simulink model "HvacActrPosnReqFrnt"  (R2022b)
settings: Solver=FixedStepDiscrete  StartTime=0.0  StopTime=10.0  FixedStep=0.2  RelTol=1e-3
(part 2 of 2)

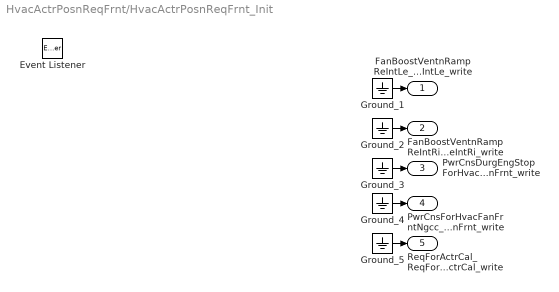
== system HvacActrPosnReqFrnt/HvacActrPosnReqFrnt_Init ==
  Event Listener : EventListener  EventName=reset
  Ground_{1..5} (5 alike) : Ground
  FanBoostVentnRampReIntLe_FanBoostVentnRampReIntLe_write : Outport
  FanBoostVentnRampReIntRi_FanBoostVentnRampReIntRi_write : Outport  Port=2
  PwrCnsDurgEngStopForHvacFanFrnt_PwrCnsDurgEngStopForHvacFanFrnt_write : Outport  Port=3
  PwrCnsForHvacFanFrntNgcc_PwrCnsForHvacFanFrnt_write : Outport  Port=4
  ReqForActrCal_ReqForActrCal_write : Outport  Port=5
  wires:
  Ground_1/1 -> FanBoostVentnRampReIntLe_FanBoostVentnRampReIntLe_write/1
  Ground_2/1 -> FanBoostVentnRampReIntRi_FanBoostVentnRampReIntRi_write/1
  Ground_3/1 -> PwrCnsDurgEngStopForHvacFanFrnt_PwrCnsDurgEngStopForHvacFanFrnt_write/1
  Ground_4/1 -> PwrCnsForHvacFanFrntNgcc_PwrCnsForHvacFanFrnt_write/1
  Ground_5/1 -> ReqForActrCal_ReqForActrCal_write/1

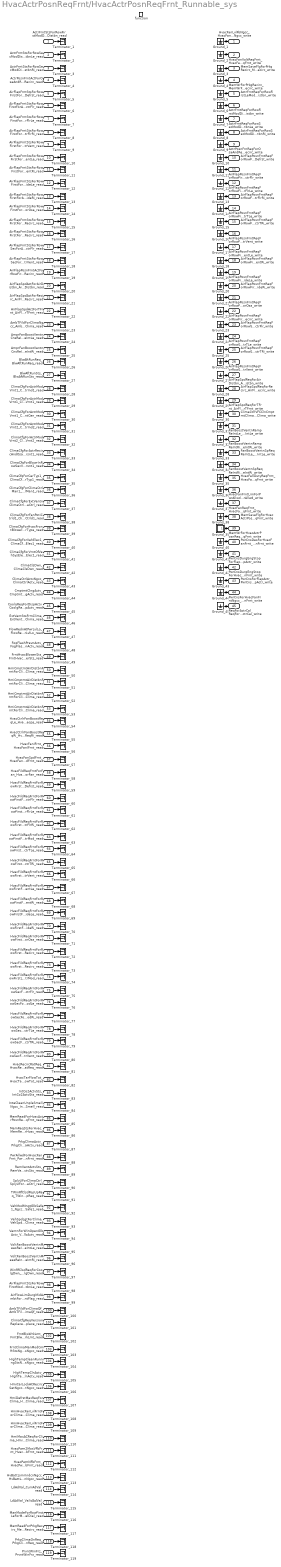
== system HvacActrPosnReqFrnt/HvacActrPosnReqFrnt_Runnable_sys ==
  ActrFrntStsForRowFirstModDistbn_ActrFrntStsForRowFirstModDistbn_read : Inport
  ActrFrntStsForRowSecModDistbnLe_ActrFrntStsForRowSecModDistbnLe_read : Inport  Port=2
  ActrFrntStsForRowSecModDistbnRi_ActrFrntStsForRowSecModDistbnRi_read : Inport  Port=3
  ActrPosnFrntActForOsaAndRecirc_ActrPosnFrntActForOsaAndRecirc_read : Inport  Port=4
  AirFlapFrntStsForRowFirstForActrDefrst_AirFlapFrntStsForRowFirstForActrDefrst_read : Inport  Port=5
  AirFlapFrntStsForRowFirstForActrFlr_AirFlapFrntStsForRowFirstForActrFlr_read : Inport  Port=6
  AirFlapFrntStsForRowFirstForActrFlrLe_AirFlapFrntStsForRowFirstForActrFlrLe_read : Inport  Port=7
  AirFlapFrntStsForRowFirstForActrFlrRi_AirFlapFrntStsForRowFirstForActrFlrRi_read : Inport  Port=8
  AirFlapFrntStsForRowFirstForActrVent_AirFlapFrntStsForRowFirstForActrVent_read : Inport  Port=9
  AirFlapFrntStsForRowFirstForActrVentLe_AirFlapFrntStsForRowFirstForActrVentLe_read : Inport  Port=10
  AirFlapFrntStsForRowFirstForActrVentRi_AirFlapFrntStsForRowFirstForActrVentRi_read : Inport  Port=11
  AirFlapFrntStsForRowFirstForActrVentSideLe_AirFlapFrntStsForRowFirstForActrVentSideLe_read : Inport  Port=12
  AirFlapFrntStsForRowFirstForActrVentSideRi_AirFlapFrntStsForRowFirstForActrVentSideRi_read : Inport  Port=13
  AirFlapFrntStsForRowFirstForOsa_AirFlapFrntStsForRowFirstForOsa_read : Inport  Port=14
  AirFlapFrntStsForRowFirstForOsaAndRecirc_AirFlapFrntStsForRowFirstForOsaAndRecirc_read : Inport  Port=15
  AirFlapFrntStsForRowFirstForRecirc_AirFlapFrntStsForRowFirstForRecirc_read : Inport  Port=16
  AirFlapFrntStsForRowSecForActrFlr_AirFlapFrntStsForRowSecForActrFlr_read : Inport  Port=17
  AirFlapFrntStsForRowSecForActrVent_AirFlapFrntStsForRowSecForActrVent_read : Inport  Port=18
  AirFlapPosnFrntActForRowFirstForRecirc_AirFlapPosnFrntActForRowFirstForRecirc_read : Inport  Port=19
  AirFlapSpdActForAirDistbn_AirFlapSpdActForAirDistbn_read : Inport  Port=20
  AirFlapSpdActForRecirc_AirFlapSpdActForRecirc_read : Inport  Port=21
  AirFlapSpdActForTFrnt_AirFlapSpdActForTFrnt_read : Inport  Port=22
  AmbTFildForClimaNgcc_AmbTFildForClima_read : Inport  Port=23
  AmprFanBoostVentnCnsReIntLe_AmprFanBoostVentnCnsReIntLe_read : Inport  Port=24
  AmprFanBoostVentnCnsReIntRi_AmprFanBoostVentnCnsReIntRi_read : Inport  Port=25
  BlwAftRunReq_BlwAftRunReq_read : Inport  Port=26
  BlwAftRunSts_BlwAftRunSts_read : Inport  Port=27
  ClimaCfgForActrModVrnt1_ClimaCfgForActrInd1_read : Inport  Port=28
  ClimaCfgForActrModVrnt1_ClimaCfgForActrModVrnt1_read : Inport  Port=29
  ClimaCfgForActrModVrnt1_ClimaCfgForActrModVrntCen_read : Inport  Port=30
  ClimaCfgForActrModVrnt2_ClimaCfgForActrInd2_read : Inport  Port=31
  ClimaCfgForActrModVrnt2_ClimaCfgForActrModVrnt2_read : Inport  Port=32
  ClimaCfgForActrRecircAndOsaCmnPrsnt1_ClimaCfgForActrRecircAndOsaCmnPrsnt1_read : Inport  Port=33
  ClimaCfgForBlowrInRowSecVentSidePrsnt1_ClimaCfgForBlowrInRowSecVentSidePrsnt1_read : Inport  Port=34
  ClimaCfgForCarTyp1_ClimaCfgForCarTyp1_read : Inport  Port=35
  ClimaCfgForClimaCtrlMan1_ClimaCfgForClimaCtrlMan1_read : Inport  Port=36
  ClimaCfgForExtVentnClimaCtrl_ClimaCfgForExtVentnClimaCtrl_read : Inport  Port=37
  ClimaCfgForFanPotiCtrld1_ClimaCfgForFanPotiCtrld1_read : Inport  Port=38
  ClimaCfgForHvacFrontBlowerType_ClimaCfgForHvacFrontBlowerType_read : Inport  Port=39
  ClimaCfgForVehElec1_ClimaCfgForVehElec1_read : Inport  Port=40
  ClimaCfgForVrntOfVehSysElec1_ClimaCfgForVrntOfVehSysElec1_read : Inport  Port=41
  ClimaClsDwn_ClimaClsDwn_read : Inport  Port=42
  ClimaCtrlActvNgcc_ClimaCtrlActv_read : Inport  Port=43
  CmptmtClngActv_CmptmtClngActv_read : Inport  Port=44
  CoolgReqForDispActv_CoolgReqForDispActv_read : Inport  Port=45
  ExtVentStsFrmClima_ExtVentStsFrmClima_read : Inport  Port=46
  FlowRednAtPwrLvlLo_FlowRednAtPwrLvlLo_read : Inport  Port=47
  FogFlashPrevnActv_FogFlashPrevnActv_read : Inport  Port=48
  FrntHvacBlowerSts_FrntHvacBlowerSts_read : Inport  Port=49
  HmiCmptmtAirDistbnFrntForClima_HmiCmptmtAirDistbnFrntForClima_read : Inport  Port=50
  HmiCmptmtAirDistbnFrntForClima_HmiCmptmtAirDistbnFrntRiForClima_read : Inport  Port=51
  HmiCmptmtAirDistbnFrntForClima_HmiCmptmtAirDistbnReForClima_read : Inport  Port=52
  HmiCmptmtAirDistbnFrntForClima_HmiCmptmtAirDistbnReRiForClima_read : Inport  Port=53
  HvacCtrlrFanBoostReqLe_HvacCtrlrFanBoostReqLe_read : Inport  Port=54
  HvacCtrlrFanBoostReqRi_HvacCtrlrFanBoostReqRi_read : Inport  Port=55
  HvacFanIFrnt_HvacFanIFrnt_read : Inport  Port=56
  HvacFanSpdFrnt_HvacFanSpdFrnt_read : Inport  Port=57
  HvacFildReqFrntForFan_HvacFildReqFrntForFan_read : Inport  Port=58
  HvacFildReqFrntForRowFirstForActrDefrst_HvacFildReqFrntForRowFirstForActrDefrst_read : Inport  Port=59
  HvacFildReqFrntForRowFirstForActrFlr_HvacFildReqFrntForRowFirstForActrFlr_read : Inport  Port=60
  HvacFildReqFrntForRowFirstForActrFlr_HvacFildReqFrntForRowFirstForActrFlrLe_read : Inport  Port=61
  HvacFildReqFrntForRowFirstForActrFlr_HvacFildReqFrntForRowFirstForActrFlrRi_read : Inport  Port=62
  HvacFildReqFrntForRowFirstForActrMod_HvacFildReqFrntForRowFirstForActrMod_read : Inport  Port=63
  HvacFildReqFrntForRowFirstForActrTLe_HvacFildReqFrntForRowFirstForActrTLe_read : Inport  Port=64
  HvacFildReqFrntForRowFirstForActrTRi_HvacFildReqFrntForRowFirstForActrTRi_read : Inport  Port=65
  HvacFildReqFrntForRowFirstForActrVent_HvacFildReqFrntForRowFirstForActrVent_read : Inport  Port=66
  HvacFildReqFrntForRowFirstForActrVent_HvacFildReqFrntForRowFirstForActrVentLe_read : Inport  Port=67
  HvacFildReqFrntForRowFirstForActrVent_HvacFildReqFrntForRowFirstForActrVentRi_read : Inport  Port=68
  HvacFildReqFrntForRowFirstForActrVent_HvacFildReqFrntForRowFirstForActrVentSideLe_read : Inport  Port=69
  HvacFildReqFrntForRowFirstForActrVent_HvacFildReqFrntForRowFirstForActrVentSideRi_read : Inport  Port=70
  HvacFildReqFrntForRowFirstForOsa_HvacFildReqFrntForRowFirstForOsa_read : Inport  Port=71
  HvacFildReqFrntForRowFirstForOsaAndRecirc_HvacFildReqFrntForRowFirstForOsaAndRecirc_read : Inport  Port=72
  HvacFildReqFrntForRowFirstForRecirc_HvacFildReqFrntForRowFirstForRecirc_read : Inport  Port=73
  HvacFildReqFrntForRowFirstLeForActrMod_HvacFildReqFrntForRowFirstLeForActrMod_read : Inport  Port=74
  HvacFildReqFrntForRowSecForActrFlr_HvacFildReqFrntForRowSecForActrFlr_read : Inport  Port=75
  HvacFildReqFrntForRowSecForActrModLe_HvacFildReqFrntForRowSecForActrModLe_read : Inport  Port=76
  HvacFildReqFrntForRowSecForActrModRi_HvacFildReqFrntForRowSecForActrModRi_read : Inport  Port=77
  HvacFildReqFrntForRowSecForActrTLe_HvacFildReqFrntForRowSecForActrTLe_read : Inport  Port=78
  HvacFildReqFrntForRowSecForActrTRi_HvacFildReqFrntForRowSecForActrTRi_read : Inport  Port=79
  HvacFildReqFrntForRowSecForActrVent_HvacFildReqFrntForRowSecForActrVent_read : Inport  Port=80
  HvacRecircRatReq_HvacRecircRatReq_read : Inport  Port=81
  HvacTarFlowTot_HvacTarFlowTot_read : Inport  Port=82
  IntCo2ActvSts_IntCo2ActvSts_read : Inport  Port=83
  InteCleanUnpleSmell_Ngcc_InteCleanUnpleSmell_read : Inport  Port=84
  MemReadForHvacActrPosnReqFrnt_MemReadForHvacActrPosnReqFrnt_read : Inport  Port=85
  MemReqStsForHvac_MemReqStsForHvac_read : Inport  Port=86
  PrkgClimaActv_PrkgClimaActv_read : Inport  Port=87
  PwrAllwdForHvacFanFrnt_PwrAllwdForHvacFanFrnt_read : Inport  Port=88
  RemVentActvSts_RemVentActvSts_read : Inport  Port=89
  SplyUForClimaCtrl_SplyUForClimaCtrl_read : Inport  Port=90
  TWinRfClsdPopUpReq_TWinRfClsdPopUpReq_read : Inport  Port=91
  VehModMngtGlbSafe1_Ngcc_VehModMngtGlbSafe1_read : Inport  Port=92
  VehSpdLgtForClima_VehSpdLgtForClima_read : Inport  Port=93
  VentnForWinOpenGlbActv_VentnForWinOpenGlbActv_read : Inport  Port=94
  VoltFanBoostVentnMeasReIntLe_VoltFanBoostVentnMeasReIntLe_read : Inport  Port=95
  VoltFanBoostVentnMeasReIntRi_VoltFanBoostVentnMeasReIntRi_read : Inport  Port=96
  WinRfClsdReqForCoolgDwn_WinRfClsdReqForCoolgDwn_read : Inport  Port=97
  AirFlapFrntStsForRowFirstModDistbnLe_AirFlapFrntStsForRowFirstModDistbnLe_read : Inport  Port=98
  AirFlowLimDurgMidAmbtForNVHForHVACFrntFlag_AirFlowLimDurgMidAmbtForNVHForHVACFrntFlag_read : Inport  Port=99
  AmbTFildForClimaQf_AmbTFildForClimaQf_read : Inport  Port=100
  ClimaCfgReqVersionReplace_ClimaCfgReqVersionReplace_read : Inport  Port=101
  FrntBlwWrkLmt_FrntBlwWrkLmt_read : Inport  Port=102
  FrntClimaManModCmftStsNgcc_FrntClimaManModCmftStsNgcc_read : Inport  Port=103
  HighTempCleanRunningStsNgcc_HighTempCleanRunningStsNgcc_read : Inport  Port=104
  HighTempClnActv_HighTempClnActv_read : Inport  Port=105
  HmiCarLockACRecircSetNgcc_HmiCarLockACRecircSetNgcc_read : Inport  Port=106
  HmiDefrstMaxReqForClima_HmiDefrstMaxReqForClima_read : Inport  Port=107
  HmiHvacFanLvlFrntForClima_HmiHvacFanLvlFrntForClima_read : Inport  Port=108
  HmiHvacFanLvlFrntForClima_HmiHvacFanLvlFrntRiForClima_read : Inport  Port=109
  HmiMaxACReqForClima_HmiMaxACReqForClima_read : Inport  Port=110
  HvacPwm2MosVFbFrnt_HvacPwm2MosVFbFrnt_read : Inport  Port=111
  HvacPwmNFbFrnt_HvacPwmNFbFrnt_read : Inport  Port=112
  HvBattLimnIndcnNgcc_HvBattLimnIndcnNgcc_read : Inport  Port=113
  LdAdVal_CurrAdVal_read : Inport  Port=114
  LdAdVal_ValidAdVal_read : Inport  Port=115
  ManModeForRowFirstLeForModelDial_ManModeForRowFirstLeForModelDial_read : Inport  Port=116
  MemReadForPrkgRecirc_MemReadForPrkgRecirc_read : Inport  Port=117
  PrkgClimaOnReq_PrkgClimaOnReq_read : Inport  Port=118
  PrvntWinFrz_PrvntWinFrz_read : Inport  Port=119
  function : TriggerPort  TriggerType=function-call  VariantControl=(inherit)  SampleTimeType=periodic  SampleTime=-1
  Ground_{1..38,40..45} (44 alike) : Ground
  Ground_39 : Subsystem
  Terminator_{1..119} (119 alike) : Terminator
  HvacFanLvlFbNgcc_HvacFanLvlFbNgcc_write : Outport
      settings: EnsureOutportIsVirtual=on
  HvacFanVoltReqFrnt_HvacFanVoltReqFrnt_write : Outport  Port=2
      settings: EnsureOutportIsVirtual=on
  MemSaveFlgForPrkgRecirc_MemSaveFlgForPrkgRecirc_write : Outport  Port=3
      settings: EnsureOutportIsVirtual=on
  MemWrForPrkgRecirc_MemWrForPrkgRecirc_write : Outport  Port=4
      settings: EnsureOutportIsVirtual=on
  ActrFrntReqForRowFirstLeModDistbn_ActrFrntReqForRowFirstLeModDistbn_write : Outport  Port=5
      settings: EnsureOutportIsVirtual=on
  ActrFrntReqForRowFirstModDistbn_ActrFrntReqForRowFirstModDistbn_write : Outport  Port=6
      settings: EnsureOutportIsVirtual=on
  ActrFrntReqForRowSecModDistbnLe_ActrFrntReqForRowSecModDistbnLe_write : Outport  Port=7
      settings: EnsureOutportIsVirtual=on
  ActrFrntReqForRowSecModDistbnRi_ActrFrntReqForRowSecModDistbnRi_write : Outport  Port=8
      settings: EnsureOutportIsVirtual=on
  ActrPosnFrnReqForOsaAndRecirc_ActrPosnFrnReqForOsaAndRecirc_write : Outport  Port=9
      settings: EnsureOutportIsVirtual=on
  AirFlapPosnFrntReqForRowFirstForActrDefrst_AirFlapPosnFrntReqForRowFirstForActrDefrst_write : Outport  Port=10
      settings: EnsureOutportIsVirtual=on
  AirFlapPosnFrntReqForRowFirstForActrFlr_AirFlapPosnFrntReqForRowFirstForActrFlr_write : Outport  Port=11
      settings: EnsureOutportIsVirtual=on
  AirFlapPosnFrntReqForRowFirstForActrFlrLe_AirFlapPosnFrntReqForRowFirstForActrFlrLe_write : Outport  Port=12
      settings: EnsureOutportIsVirtual=on
  AirFlapPosnFrntReqForRowFirstForActrFlrRi_AirFlapPosnFrntReqForRowFirstForActrFlrRi_write : Outport  Port=13
      settings: EnsureOutportIsVirtual=on
  AirFlapPosnFrntReqForRowFirstForActrTLe_AirFlapPosnFrntReqForRowFirstForActrTLe_write : Outport  Port=14
      settings: EnsureOutportIsVirtual=on
  AirFlapPosnFrntReqForRowFirstForActrTRi_AirFlapPosnFrntReqForRowFirstForActrTRi_write : Outport  Port=15
      settings: EnsureOutportIsVirtual=on
  AirFlapPosnFrntReqForRowFirstForActrVent_AirFlapPosnFrntReqForRowFirstForActrVent_write : Outport  Port=16
      settings: EnsureOutportIsVirtual=on
  AirFlapPosnFrntReqForRowFirstForActrVentLe_AirFlapPosnFrntReqForRowFirstForActrVentLe_write : Outport  Port=17
      settings: EnsureOutportIsVirtual=on
  AirFlapPosnFrntReqForRowFirstForActrVentRi_AirFlapPosnFrntReqForRowFirstForActrVentRi_write : Outport  Port=18
      settings: EnsureOutportIsVirtual=on
  AirFlapPosnFrntReqForRowFirstForActrVentSideLe_AirFlapPosnFrntReqForRowFirstForActrVentSideLe_write : Outport  Port=19
      settings: EnsureOutportIsVirtual=on
  AirFlapPosnFrntReqForRowFirstForActrVentSideRi_AirFlapPosnFrntReqForRowFirstForActrVentSideRi_write : Outport  Port=20
      settings: EnsureOutportIsVirtual=on
  AirFlapPosnFrntReqForRowFirstForOsa_AirFlapPosnFrntReqForRowFirstForOsa_write : Outport  Port=21
      settings: EnsureOutportIsVirtual=on
  AirFlapPosnFrntReqForRowFirstForRecirc_AirFlapPosnFrntReqForRowFirstForRecirc_write : Outport  Port=22
      settings: EnsureOutportIsVirtual=on
  AirFlapPosnFrntReqForRowSecForActrFlr_AirFlapPosnFrntReqForRowSecForActrFlr_write : Outport  Port=23
      settings: EnsureOutportIsVirtual=on
  AirFlapPosnFrntReqForRowSecForActrTLe_AirFlapPosnFrntReqForRowSecForActrTLe_write : Outport  Port=24
      settings: EnsureOutportIsVirtual=on
  AirFlapPosnFrntReqForRowSecForActrTRi_AirFlapPosnFrntReqForRowSecForActrTRi_write : Outport  Port=25
      settings: EnsureOutportIsVirtual=on
  AirFlapPosnFrntReqForRowSecForActrVent_AirFlapPosnFrntReqForRowSecForActrVent_write : Outport  Port=26
      settings: EnsureOutportIsVirtual=on
  AirFlapSpdReqForAirDistbn_AirFlapSpdReqForAirDistbn_write : Outport  Port=27
      settings: EnsureOutportIsVirtual=on
  AirFlapSpdReqForRecirc_AirFlapSpdReqForRecirc_write : Outport  Port=28
      settings: EnsureOutportIsVirtual=on
  AirFlapSpdReqForTFrnt_AirFlapSpdReqForTFrnt_write : Outport  Port=29
      settings: EnsureOutportIsVirtual=on
  ClimaCtrlVFCClnCmptmtClima_ClimaCtrlVFCClnCmptmtClima_write : Outport  Port=30
      settings: EnsureOutportIsVirtual=on
  FanBoostVentnRampReIntLe_FanBoostVentnRampReIntLe_write : Outport  Port=31
      settings: EnsureOutportIsVirtual=on
  FanBoostVentnRampReIntRi_FanBoostVentnRampReIntRi_write : Outport  Port=32
      settings: EnsureOutportIsVirtual=on
  FanBoostVentnSpReqReIntLe_FanBoostVentnSpReqReIntLe_write : Outport  Port=33
      settings: EnsureOutportIsVirtual=on
  FanBoostVentnSpReqReIntRi_FanBoostVentnSpReqReIntRi_write : Outport  Port=34
      settings: EnsureOutportIsVirtual=on
  HvacFanDutyReqFrnt_HvacFanDutyReqFrnt_write : Outport  Port=35
      settings: EnsureOutportIsVirtual=on
  HvacFanFrntLimForPwrAllwd_HvacFanFrntLimForPwrAllwd_write : Outport  Port=36
      settings: EnsureOutportIsVirtual=on
  HvacFanReqFrnt_HvacFanReqFrnt_write : Outport  Port=37
      settings: EnsureOutportIsVirtual=on
  MemSaveFlgForHvacActrPosnReqFrnt_MemSaveFlgForHvacActrPosnReqFrnt_write : Outport  Port=38
      settings: EnsureOutportIsVirtual=on
  MemWrForHvacActrPosnReqFrnt_MemWrForHvacActrPosnReqFrnt_write : Outport  Port=39
      settings: EnsureOutportIsVirtual=on
  PwrCnsDesForHvacFanFrnt_PwrCnsDesForHvacFanFrnt_write : Outport  Port=40
      settings: EnsureOutportIsVirtual=on
  PwrCnsDurgEngStopForFlapActr_PwrCnsDurgEngStopForFlapActr_write : Outport  Port=41
      settings: EnsureOutportIsVirtual=on
  PwrCnsDurgEngStopForHvacFanFrnt_PwrCnsDurgEngStopForHvacFanFrnt_write : Outport  Port=42
      settings: EnsureOutportIsVirtual=on
  PwrCnsForFlapActr_PwrCnsForFlapActr_write : Outport  Port=43
      settings: EnsureOutportIsVirtual=on
  PwrCnsForHvacFanFrntNgcc_PwrCnsForHvacFanFrnt_write : Outport  Port=44
      settings: EnsureOutportIsVirtual=on
  ReqForActrCal_ReqForActrCal_write : Outport  Port=45
      settings: EnsureOutportIsVirtual=on
  wires:
  ActrFrntStsForRowFirstModDistbn_ActrFrntStsForRowFirstModDistbn_read/1 -> Terminator_1/1
  ActrFrntStsForRowSecModDistbnLe_ActrFrntStsForRowSecModDistbnLe_read/1 -> Terminator_2/1
  ActrFrntStsForRowSecModDistbnRi_ActrFrntStsForRowSecModDistbnRi_read/1 -> Terminator_3/1
  ActrPosnFrntActForOsaAndRecirc_ActrPosnFrntActForOsaAndRecirc_read/1 -> Terminator_4/1
  AirFlapFrntStsForRowFirstForActrDefrst_AirFlapFrntStsForRowFirstForActrDefrst_read/1 -> Terminator_5/1
  AirFlapFrntStsForRowFirstForActrFlr_AirFlapFrntStsForRowFirstForActrFlr_read/1 -> Terminator_6/1
  AirFlapFrntStsForRowFirstForActrFlrLe_AirFlapFrntStsForRowFirstForActrFlrLe_read/1 -> Terminator_7/1
  AirFlapFrntStsForRowFirstForActrFlrRi_AirFlapFrntStsForRowFirstForActrFlrRi_read/1 -> Terminator_8/1
  AirFlapFrntStsForRowFirstForActrVent_AirFlapFrntStsForRowFirstForActrVent_read/1 -> Terminator_9/1
  AirFlapFrntStsForRowFirstForActrVentLe_AirFlapFrntStsForRowFirstForActrVentLe_read/1 -> Terminator_10/1
  AirFlapFrntStsForRowFirstForActrVentRi_AirFlapFrntStsForRowFirstForActrVentRi_read/1 -> Terminator_11/1
  AirFlapFrntStsForRowFirstForActrVentSideLe_AirFlapFrntStsForRowFirstForActrVentSideLe_read/1 -> Terminator_12/1
  AirFlapFrntStsForRowFirstForActrVentSideRi_AirFlapFrntStsForRowFirstForActrVentSideRi_read/1 -> Terminator_13/1
  AirFlapFrntStsForRowFirstForOsa_AirFlapFrntStsForRowFirstForOsa_read/1 -> Terminator_14/1
  AirFlapFrntStsForRowFirstForOsaAndRecirc_AirFlapFrntStsForRowFirstForOsaAndRecirc_read/1 -> Terminator_15/1
  AirFlapFrntStsForRowFirstForRecirc_AirFlapFrntStsForRowFirstForRecirc_read/1 -> Terminator_16/1
  AirFlapFrntStsForRowSecForActrFlr_AirFlapFrntStsForRowSecForActrFlr_read/1 -> Terminator_17/1
  AirFlapFrntStsForRowSecForActrVent_AirFlapFrntStsForRowSecForActrVent_read/1 -> Terminator_18/1
  AirFlapPosnFrntActForRowFirstForRecirc_AirFlapPosnFrntActForRowFirstForRecirc_read/1 -> Terminator_19/1
  AirFlapSpdActForAirDistbn_AirFlapSpdActForAirDistbn_read/1 -> Terminator_20/1
  AirFlapSpdActForRecirc_AirFlapSpdActForRecirc_read/1 -> Terminator_21/1
  AirFlapSpdActForTFrnt_AirFlapSpdActForTFrnt_read/1 -> Terminator_22/1
  AmbTFildForClimaNgcc_AmbTFildForClima_read/1 -> Terminator_23/1
  AmprFanBoostVentnCnsReIntLe_AmprFanBoostVentnCnsReIntLe_read/1 -> Terminator_24/1
  AmprFanBoostVentnCnsReIntRi_AmprFanBoostVentnCnsReIntRi_read/1 -> Terminator_25/1
  BlwAftRunReq_BlwAftRunReq_read/1 -> Terminator_26/1
  BlwAftRunSts_BlwAftRunSts_read/1 -> Terminator_27/1
  ClimaCfgForActrModVrnt1_ClimaCfgForActrInd1_read/1 -> Terminator_28/1
  ClimaCfgForActrModVrnt1_ClimaCfgForActrModVrnt1_read/1 -> Terminator_29/1
  ClimaCfgForActrModVrnt1_ClimaCfgForActrModVrntCen_read/1 -> Terminator_30/1
  ClimaCfgForActrModVrnt2_ClimaCfgForActrInd2_read/1 -> Terminator_31/1
  ClimaCfgForActrModVrnt2_ClimaCfgForActrModVrnt2_read/1 -> Terminator_32/1
  ClimaCfgForActrRecircAndOsaCmnPrsnt1_ClimaCfgForActrRecircAndOsaCmnPrsnt1_read/1 -> Terminator_33/1
  ClimaCfgForBlowrInRowSecVentSidePrsnt1_ClimaCfgForBlowrInRowSecVentSidePrsnt1_read/1 -> Terminator_34/1
  ClimaCfgForCarTyp1_ClimaCfgForCarTyp1_read/1 -> Terminator_35/1
  ClimaCfgForClimaCtrlMan1_ClimaCfgForClimaCtrlMan1_read/1 -> Terminator_36/1
  ClimaCfgForExtVentnClimaCtrl_ClimaCfgForExtVentnClimaCtrl_read/1 -> Terminator_37/1
  ClimaCfgForFanPotiCtrld1_ClimaCfgForFanPotiCtrld1_read/1 -> Terminator_38/1
  ClimaCfgForHvacFrontBlowerType_ClimaCfgForHvacFrontBlowerType_read/1 -> Terminator_39/1
  ClimaCfgForVehElec1_ClimaCfgForVehElec1_read/1 -> Terminator_40/1
  ClimaCfgForVrntOfVehSysElec1_ClimaCfgForVrntOfVehSysElec1_read/1 -> Terminator_41/1
  ClimaClsDwn_ClimaClsDwn_read/1 -> Terminator_42/1
  ClimaCtrlActvNgcc_ClimaCtrlActv_read/1 -> Terminator_43/1
  CmptmtClngActv_CmptmtClngActv_read/1 -> Terminator_44/1
  CoolgReqForDispActv_CoolgReqForDispActv_read/1 -> Terminator_45/1
  ExtVentStsFrmClima_ExtVentStsFrmClima_read/1 -> Terminator_46/1
  FlowRednAtPwrLvlLo_FlowRednAtPwrLvlLo_read/1 -> Terminator_47/1
  FogFlashPrevnActv_FogFlashPrevnActv_read/1 -> Terminator_48/1
  FrntHvacBlowerSts_FrntHvacBlowerSts_read/1 -> Terminator_49/1
  HmiCmptmtAirDistbnFrntForClima_HmiCmptmtAirDistbnFrntForClima_read/1 -> Terminator_50/1
  HmiCmptmtAirDistbnFrntForClima_HmiCmptmtAirDistbnFrntRiForClima_read/1 -> Terminator_51/1
  HmiCmptmtAirDistbnFrntForClima_HmiCmptmtAirDistbnReForClima_read/1 -> Terminator_52/1
  HmiCmptmtAirDistbnFrntForClima_HmiCmptmtAirDistbnReRiForClima_read/1 -> Terminator_53/1
  HvacCtrlrFanBoostReqLe_HvacCtrlrFanBoostReqLe_read/1 -> Terminator_54/1
  HvacCtrlrFanBoostReqRi_HvacCtrlrFanBoostReqRi_read/1 -> Terminator_55/1
  HvacFanIFrnt_HvacFanIFrnt_read/1 -> Terminator_56/1
  HvacFanSpdFrnt_HvacFanSpdFrnt_read/1 -> Terminator_57/1
  HvacFildReqFrntForFan_HvacFildReqFrntForFan_read/1 -> Terminator_58/1
  HvacFildReqFrntForRowFirstForActrDefrst_HvacFildReqFrntForRowFirstForActrDefrst_read/1 -> Terminator_59/1
  HvacFildReqFrntForRowFirstForActrFlr_HvacFildReqFrntForRowFirstForActrFlr_read/1 -> Terminator_60/1
  HvacFildReqFrntForRowFirstForActrFlr_HvacFildReqFrntForRowFirstForActrFlrLe_read/1 -> Terminator_61/1
  HvacFildReqFrntForRowFirstForActrFlr_HvacFildReqFrntForRowFirstForActrFlrRi_read/1 -> Terminator_62/1
  HvacFildReqFrntForRowFirstForActrMod_HvacFildReqFrntForRowFirstForActrMod_read/1 -> Terminator_63/1
  HvacFildReqFrntForRowFirstForActrTLe_HvacFildReqFrntForRowFirstForActrTLe_read/1 -> Terminator_64/1
  HvacFildReqFrntForRowFirstForActrTRi_HvacFildReqFrntForRowFirstForActrTRi_read/1 -> Terminator_65/1
  HvacFildReqFrntForRowFirstForActrVent_HvacFildReqFrntForRowFirstForActrVent_read/1 -> Terminator_66/1
  HvacFildReqFrntForRowFirstForActrVent_HvacFildReqFrntForRowFirstForActrVentLe_read/1 -> Terminator_67/1
  HvacFildReqFrntForRowFirstForActrVent_HvacFildReqFrntForRowFirstForActrVentRi_read/1 -> Terminator_68/1
  HvacFildReqFrntForRowFirstForActrVent_HvacFildReqFrntForRowFirstForActrVentSideLe_read/1 -> Terminator_69/1
  HvacFildReqFrntForRowFirstForActrVent_HvacFildReqFrntForRowFirstForActrVentSideRi_read/1 -> Terminator_70/1
  HvacFildReqFrntForRowFirstForOsa_HvacFildReqFrntForRowFirstForOsa_read/1 -> Terminator_71/1
  HvacFildReqFrntForRowFirstForOsaAndRecirc_HvacFildReqFrntForRowFirstForOsaAndRecirc_read/1 -> Terminator_72/1
  HvacFildReqFrntForRowFirstForRecirc_HvacFildReqFrntForRowFirstForRecirc_read/1 -> Terminator_73/1
  HvacFildReqFrntForRowFirstLeForActrMod_HvacFildReqFrntForRowFirstLeForActrMod_read/1 -> Terminator_74/1
  HvacFildReqFrntForRowSecForActrFlr_HvacFildReqFrntForRowSecForActrFlr_read/1 -> Terminator_75/1
  HvacFildReqFrntForRowSecForActrModLe_HvacFildReqFrntForRowSecForActrModLe_read/1 -> Terminator_76/1
  HvacFildReqFrntForRowSecForActrModRi_HvacFildReqFrntForRowSecForActrModRi_read/1 -> Terminator_77/1
  HvacFildReqFrntForRowSecForActrTLe_HvacFildReqFrntForRowSecForActrTLe_read/1 -> Terminator_78/1
  HvacFildReqFrntForRowSecForActrTRi_HvacFildReqFrntForRowSecForActrTRi_read/1 -> Terminator_79/1
  HvacFildReqFrntForRowSecForActrVent_HvacFildReqFrntForRowSecForActrVent_read/1 -> Terminator_80/1
  HvacRecircRatReq_HvacRecircRatReq_read/1 -> Terminator_81/1
  HvacTarFlowTot_HvacTarFlowTot_read/1 -> Terminator_82/1
  IntCo2ActvSts_IntCo2ActvSts_read/1 -> Terminator_83/1
  InteCleanUnpleSmell_Ngcc_InteCleanUnpleSmell_read/1 -> Terminator_84/1
  MemReadForHvacActrPosnReqFrnt_MemReadForHvacActrPosnReqFrnt_read/1 -> Terminator_85/1
  MemReqStsForHvac_MemReqStsForHvac_read/1 -> Terminator_86/1
  PrkgClimaActv_PrkgClimaActv_read/1 -> Terminator_87/1
  PwrAllwdForHvacFanFrnt_PwrAllwdForHvacFanFrnt_read/1 -> Terminator_88/1
  RemVentActvSts_RemVentActvSts_read/1 -> Terminator_89/1
  SplyUForClimaCtrl_SplyUForClimaCtrl_read/1 -> Terminator_90/1
  TWinRfClsdPopUpReq_TWinRfClsdPopUpReq_read/1 -> Terminator_91/1
  VehModMngtGlbSafe1_Ngcc_VehModMngtGlbSafe1_read/1 -> Terminator_92/1
  VehSpdLgtForClima_VehSpdLgtForClima_read/1 -> Terminator_93/1
  VentnForWinOpenGlbActv_VentnForWinOpenGlbActv_read/1 -> Terminator_94/1
  VoltFanBoostVentnMeasReIntLe_VoltFanBoostVentnMeasReIntLe_read/1 -> Terminator_95/1
  VoltFanBoostVentnMeasReIntRi_VoltFanBoostVentnMeasReIntRi_read/1 -> Terminator_96/1
  WinRfClsdReqForCoolgDwn_WinRfClsdReqForCoolgDwn_read/1 -> Terminator_97/1
  AirFlapFrntStsForRowFirstModDistbnLe_AirFlapFrntStsForRowFirstModDistbnLe_read/1 -> Terminator_98/1
  AirFlowLimDurgMidAmbtForNVHForHVACFrntFlag_AirFlowLimDurgMidAmbtForNVHForHVACFrntFlag_read/1 -> Terminator_99/1
  AmbTFildForClimaQf_AmbTFildForClimaQf_read/1 -> Terminator_100/1
  ClimaCfgReqVersionReplace_ClimaCfgReqVersionReplace_read/1 -> Terminator_101/1
  FrntBlwWrkLmt_FrntBlwWrkLmt_read/1 -> Terminator_102/1
  FrntClimaManModCmftStsNgcc_FrntClimaManModCmftStsNgcc_read/1 -> Terminator_103/1
  HighTempCleanRunningStsNgcc_HighTempCleanRunningStsNgcc_read/1 -> Terminator_104/1
  HighTempClnActv_HighTempClnActv_read/1 -> Terminator_105/1
  HmiCarLockACRecircSetNgcc_HmiCarLockACRecircSetNgcc_read/1 -> Terminator_106/1
  HmiDefrstMaxReqForClima_HmiDefrstMaxReqForClima_read/1 -> Terminator_107/1
  HmiHvacFanLvlFrntForClima_HmiHvacFanLvlFrntForClima_read/1 -> Terminator_108/1
  HmiHvacFanLvlFrntForClima_HmiHvacFanLvlFrntRiForClima_read/1 -> Terminator_109/1
  HmiMaxACReqForClima_HmiMaxACReqForClima_read/1 -> Terminator_110/1
  HvacPwm2MosVFbFrnt_HvacPwm2MosVFbFrnt_read/1 -> Terminator_111/1
  HvacPwmNFbFrnt_HvacPwmNFbFrnt_read/1 -> Terminator_112/1
  HvBattLimnIndcnNgcc_HvBattLimnIndcnNgcc_read/1 -> Terminator_113/1
  LdAdVal_CurrAdVal_read/1 -> Terminator_114/1
  LdAdVal_ValidAdVal_read/1 -> Terminator_115/1
  ManModeForRowFirstLeForModelDial_ManModeForRowFirstLeForModelDial_read/1 -> Terminator_116/1
  MemReadForPrkgRecirc_MemReadForPrkgRecirc_read/1 -> Terminator_117/1
  PrkgClimaOnReq_PrkgClimaOnReq_read/1 -> Terminator_118/1
  PrvntWinFrz_PrvntWinFrz_read/1 -> Terminator_119/1
  Ground_1/1 -> HvacFanLvlFbNgcc_HvacFanLvlFbNgcc_write/1
  Ground_2/1 -> HvacFanVoltReqFrnt_HvacFanVoltReqFrnt_write/1
  Ground_3/1 -> MemSaveFlgForPrkgRecirc_MemSaveFlgForPrkgRecirc_write/1
  Ground_4/1 -> MemWrForPrkgRecirc_MemWrForPrkgRecirc_write/1
  Ground_5/1 -> ActrFrntReqForRowFirstLeModDistbn_ActrFrntReqForRowFirstLeModDistbn_write/1
  Ground_6/1 -> ActrFrntReqForRowFirstModDistbn_ActrFrntReqForRowFirstModDistbn_write/1
  Ground_7/1 -> ActrFrntReqForRowSecModDistbnLe_ActrFrntReqForRowSecModDistbnLe_write/1
  Ground_8/1 -> ActrFrntReqForRowSecModDistbnRi_ActrFrntReqForRowSecModDistbnRi_write/1
  Ground_9/1 -> ActrPosnFrnReqForOsaAndRecirc_ActrPosnFrnReqForOsaAndRecirc_write/1
  Ground_10/1 -> AirFlapPosnFrntReqForRowFirstForActrDefrst_AirFlapPosnFrntReqForRowFirstForActrDefrst_write/1
  Ground_11/1 -> AirFlapPosnFrntReqForRowFirstForActrFlr_AirFlapPosnFrntReqForRowFirstForActrFlr_write/1
  Ground_12/1 -> AirFlapPosnFrntReqForRowFirstForActrFlrLe_AirFlapPosnFrntReqForRowFirstForActrFlrLe_write/1
  Ground_13/1 -> AirFlapPosnFrntReqForRowFirstForActrFlrRi_AirFlapPosnFrntReqForRowFirstForActrFlrRi_write/1
  Ground_14/1 -> AirFlapPosnFrntReqForRowFirstForActrTLe_AirFlapPosnFrntReqForRowFirstForActrTLe_write/1
  Ground_15/1 -> AirFlapPosnFrntReqForRowFirstForActrTRi_AirFlapPosnFrntReqForRowFirstForActrTRi_write/1
  Ground_16/1 -> AirFlapPosnFrntReqForRowFirstForActrVent_AirFlapPosnFrntReqForRowFirstForActrVent_write/1
  Ground_17/1 -> AirFlapPosnFrntReqForRowFirstForActrVentLe_AirFlapPosnFrntReqForRowFirstForActrVentLe_write/1
  Ground_18/1 -> AirFlapPosnFrntReqForRowFirstForActrVentRi_AirFlapPosnFrntReqForRowFirstForActrVentRi_write/1
  Ground_19/1 -> AirFlapPosnFrntReqForRowFirstForActrVentSideLe_AirFlapPosnFrntReqForRowFirstForActrVentSideLe_write/1
  Ground_20/1 -> AirFlapPosnFrntReqForRowFirstForActrVentSideRi_AirFlapPosnFrntReqForRowFirstForActrVentSideRi_write/1
  Ground_21/1 -> AirFlapPosnFrntReqForRowFirstForOsa_AirFlapPosnFrntReqForRowFirstForOsa_write/1
  Ground_22/1 -> AirFlapPosnFrntReqForRowFirstForRecirc_AirFlapPosnFrntReqForRowFirstForRecirc_write/1
  Ground_23/1 -> AirFlapPosnFrntReqForRowSecForActrFlr_AirFlapPosnFrntReqForRowSecForActrFlr_write/1
  Ground_24/1 -> AirFlapPosnFrntReqForRowSecForActrTLe_AirFlapPosnFrntReqForRowSecForActrTLe_write/1
  Ground_25/1 -> AirFlapPosnFrntReqForRowSecForActrTRi_AirFlapPosnFrntReqForRowSecForActrTRi_write/1
  Ground_26/1 -> AirFlapPosnFrntReqForRowSecForActrVent_AirFlapPosnFrntReqForRowSecForActrVent_write/1
  Ground_27/1 -> AirFlapSpdReqForAirDistbn_AirFlapSpdReqForAirDistbn_write/1
  Ground_28/1 -> AirFlapSpdReqForRecirc_AirFlapSpdReqForRecirc_write/1
  Ground_29/1 -> AirFlapSpdReqForTFrnt_AirFlapSpdReqForTFrnt_write/1
  Ground_30/1 -> ClimaCtrlVFCClnCmptmtClima_ClimaCtrlVFCClnCmptmtClima_write/1
  Ground_31/1 -> FanBoostVentnRampReIntLe_FanBoostVentnRampReIntLe_write/1
  Ground_32/1 -> FanBoostVentnRampReIntRi_FanBoostVentnRampReIntRi_write/1
  Ground_33/1 -> FanBoostVentnSpReqReIntLe_FanBoostVentnSpReqReIntLe_write/1
  Ground_34/1 -> FanBoostVentnSpReqReIntRi_FanBoostVentnSpReqReIntRi_write/1
  Ground_35/1 -> HvacFanDutyReqFrnt_HvacFanDutyReqFrnt_write/1
  Ground_36/1 -> HvacFanFrntLimForPwrAllwd_HvacFanFrntLimForPwrAllwd_write/1
  Ground_37/1 -> HvacFanReqFrnt_HvacFanReqFrnt_write/1
  Ground_38/1 -> MemSaveFlgForHvacActrPosnReqFrnt_MemSaveFlgForHvacActrPosnReqFrnt_write/1
  Ground_39/1(Out1) -> MemWrForHvacActrPosnReqFrnt_MemWrForHvacActrPosnReqFrnt_write/1
  Ground_40/1 -> PwrCnsDesForHvacFanFrnt_PwrCnsDesForHvacFanFrnt_write/1
  Ground_41/1 -> PwrCnsDurgEngStopForFlapActr_PwrCnsDurgEngStopForFlapActr_write/1
  Ground_42/1 -> PwrCnsDurgEngStopForHvacFanFrnt_PwrCnsDurgEngStopForHvacFanFrnt_write/1
  Ground_43/1 -> PwrCnsForFlapActr_PwrCnsForFlapActr_write/1
  Ground_44/1 -> PwrCnsForHvacFanFrntNgcc_PwrCnsForHvacFanFrnt_write/1
  Ground_45/1 -> ReqForActrCal_ReqForActrCal_write/1

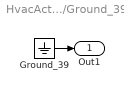
== system HvacActrPosnReqFrnt/HvacActrPosnReqFrnt_Runnable_sys/Ground_39 ==
  Ground_39 : Ground
  Out1 : Outport  OutDataTypeStr=Bus: MemRecForHvacActrPosnReqFrnt  PortDimensions=1
  wires:
  Ground_39/1 -> Out1/1
